simulink model "MX_Gateway"  (Simulink 9.0)
settings: Solver=FixedStepDiscrete  StartTime=0.0  StopTime=100  FixedStep=0.01  RelTol=1e-3
values from neighbouring files (symbols this model uses):
  ACTIVE = Bobcat.Parameter; CreateDefine( ACTIVE                         ,1                          ,'Define' ,'defines.h' )    [defines.m]
  ACTIVE_MODE_ONE = Bobcat.Parameter; CreateDefine( ACTIVE_MODE_ONE                ,19                         ,'Define' ,'defines.h' )    [defines.m]
  AUTHENTICATED = Bobcat.Parameter; CreateDefine( AUTHENTICATED                  ,1                          ,'Define' ,'defines.h' )    [defines.m]
  AUX_EMEA_CONTROLS = Bobcat.Parameter; CreateDefine( AUX_EMEA_CONTROLS  ,1   ,'Define' ,'defines.h' )    [enums.m]
  AUX_FLOW_HIGH = Bobcat.Parameter; CreateDefine( AUX_FLOW_HIGH           ,4  ,'Define' ,'defines.h' )    [enums.m]
  AUX_FLOW_INACTIVE = Bobcat.Parameter; CreateDefine( AUX_FLOW_INACTIVE       ,0  ,'Define' ,'defines.h' )    [enums.m]
  AUX_FLOW_LOW = Bobcat.Parameter; CreateDefine( AUX_FLOW_LOW            ,1  ,'Define' ,'defines.h' )    [enums.m]
  AUX_FLOW_MEDIUM = Bobcat.Parameter; CreateDefine( AUX_FLOW_MEDIUM         ,2  ,'Define' ,'defines.h' )    [enums.m]
  AUX_FLOW_STANDARD = Bobcat.Parameter; CreateDefine( AUX_FLOW_STANDARD       ,3  ,'Define' ,'defines.h' )    [enums.m]
  AUX_NON_FUNCTIONAL = Bobcat.Parameter; CreateDefine( AUX_NON_FUNCTIONAL ,255 ,'Define' ,'defines.h' )    [enums.m]
  BASE_ACTIVE = Bobcat.Parameter; CreateDefine( BASE_ACTIVE                    ,253                        ,'Define' ,'defines.h' )    [defines.m]
  BASE_DETENT = Bobcat.Parameter; CreateDefine( BASE_DETENT                    ,1                          ,'Define' ,'defines.h' )    [defines.m]
  BEEP_NONE = Bobcat.Parameter; CreateDefine( BEEP_NONE                      ,255                        ,'Define' ,'defines.h' )    [defines.m]
  CD_AIR_FILTER_DEBOUNCE_NORMAL = Bobcat.Parameter; CreateAliasCD( CD_AIR_FILTER_DEBOUNCE_NORMAL                        ,uint16(500)                ,'ConstantDataPointer' ,'CONSTANT_DATA' ,'AIR_FILTER_DEBOUNCE_NORMAL')    [cdata.m]    (also in hc12_cdata.m; m4_cdata.m)
  CD_AIR_FILTER_DEBOUNCE_NOT_CONNECTED = Bobcat.Parameter; CreateAliasCD( CD_AIR_FILTER_DEBOUNCE_NOT_CONNECTED                 ,uint16(3000)               ,'ConstantDataPointer' ,'CONSTANT_DATA' ,'AIR_FILTER_DEBOUNCE_NOT_CONNECTED')    [cdata.m]    (also in hc12_cdata.m; m4_cdata.m)
  CD_AIR_FILTER_DEBOUNCE_PLUGGED = Bobcat.Parameter; CreateAliasCD( CD_AIR_FILTER_DEBOUNCE_PLUGGED                       ,uint16(3000)               ,'ConstantDataPointer' ,'CONSTANT_DATA' ,'AIR_FILTER_DEBOUNCE_PLUGGED')    [cdata.m]    (also in hc12_cdata.m; m4_cdata.m)
  CD_AIR_FILTER_FUNCTION = Bobcat.Parameter; CreateAliasCD( CD_AIR_FILTER_FUNCTION                               ,uint8(255)                 ,'ConstantDataPointer' ,'CONSTANT_DATA' ,'AIR_FILTER_FUNCTION')    [cdata.m]    (also in hc12_cdata.m; m4_cdata.m)
  CD_AIR_FILTER_PLUGGED_WEIGHT = Bobcat.Parameter; CreateAliasCD( CD_AIR_FILTER_PLUGGED_WEIGHT                         ,uint8(2)                   ,'ConstantDataPointer' ,'CONSTANT_DATA' ,'AIR_FILTER_PLUGGED_WEIGHT')    [cdata.m]    (also in hc12_cdata.m; m4_cdata.m)
  CD_AIR_FILTER_UNPLUGGED_WEIGHT = Bobcat.Parameter; CreateAliasCD( CD_AIR_FILTER_UNPLUGGED_WEIGHT                       ,uint8(1)                   ,'ConstantDataPointer' ,'CONSTANT_DATA' ,'AIR_FILTER_UNPLUGGED_WEIGHT')    [cdata.m]    (also in hc12_cdata.m; m4_cdata.m)
  CD_AUTOIDLE_ARMBAR_DELAY = Bobcat.Parameter; CreateAliasCD( CD_AUTOIDLE_ARMBAR_DELAY                             ,uint8(2)                   ,'ConstantDataPointer' ,'CONSTANT_DATA' ,'AUTOIDLE_ARMBAR_DELAY')    [cdata.m]    (also in hc12_cdata.m; m4_cdata.m)
  CD_AUTOIDLE_FUNCTION = Bobcat.Parameter; CreateAliasCD( CD_AUTOIDLE_FUNCTION                                 ,uint8(255)                 ,'ConstantDataPointer' ,'CONSTANT_DATA' ,'AUTOIDLE_FUNCTION')    [cdata.m]    (also in hc12_cdata.m; m4_cdata.m)
  CD_AUTOIDLE_IDLE_DELAY = Bobcat.Parameter; CreateAliasCD( CD_AUTOIDLE_IDLE_DELAY                               ,uint8(40)                  ,'ConstantDataPointer' ,'CONSTANT_DATA' ,'AUTOIDLE_IDLE_DELAY')    [cdata.m]    (also in hc12_cdata.m; m4_cdata.m)
  CD_AUTOIDLE_LOW_IDLE_START = Bobcat.Parameter; CreateAliasCD( CD_AUTOIDLE_LOW_IDLE_START                           ,uint8(255)                 ,'ConstantDataPointer' ,'CONSTANT_DATA' ,'AUTOIDLE_LOW_IDLE_START')    [cdata.m]    (also in hc12_cdata.m; m4_cdata.m)
  CD_AUTOIDLE_PRESSURE_OFF = Bobcat.Parameter; CreateAliasCD( CD_AUTOIDLE_PRESSURE_OFF                             ,uint16(300)                ,'ConstantDataPointer' ,'CONSTANT_DATA' ,'AUTOIDLE_PRESSURE_OFF')    [cdata.m]    (also in hc12_cdata.m; m4_cdata.m)
  CD_AUTOIDLE_PRESSURE_ON = Bobcat.Parameter; CreateAliasCD( CD_AUTOIDLE_PRESSURE_ON                              ,uint16(270)                ,'ConstantDataPointer' ,'CONSTANT_DATA' ,'AUTOIDLE_PRESSURE_ON')    [cdata.m]    (also in hc12_cdata.m; m4_cdata.m)
  CD_AUTOSHIFT_FUNCTION = Bobcat.Parameter; CreateAliasCD( CD_AUTOSHIFT_FUNCTION                                ,uint8(255)                 ,'ConstantDataPointer' ,'CONSTANT_DATA' ,'AUTOSHIFT_FUNCTION')    [cdata.m]    (also in hc12_cdata.m; m4_cdata.m)
  CD_AUTOSHIFT_PHIGH1 = Bobcat.Parameter; CreateAliasCD( CD_AUTOSHIFT_PHIGH1                                  ,uint16(205)                ,'ConstantDataPointer' ,'CONSTANT_DATA' ,'AUTOSHIFT_PHIGH1')    [cdata.m]    (also in hc12_cdata.m; m4_cdata.m)
  CD_AUTOSHIFT_PHIGH2 = Bobcat.Parameter; CreateAliasCD( CD_AUTOSHIFT_PHIGH2                                  ,uint16(193)                ,'ConstantDataPointer' ,'CONSTANT_DATA' ,'AUTOSHIFT_PHIGH2')    [cdata.m]    (also in hc12_cdata.m; m4_cdata.m)
  CD_AUTOSHIFT_PHIGH_TEMP1 = Bobcat.Parameter; CreateAliasCD( CD_AUTOSHIFT_PHIGH_TEMP1                             ,int16(70)                  ,'ConstantDataPointer' ,'CONSTANT_DATA' ,'AUTOSHIFT_PHIGH_TEMP1')    [cdata.m]    (also in hc12_cdata.m; m4_cdata.m)
  CD_AUTOSHIFT_PHIGH_TEMP2 = Bobcat.Parameter; CreateAliasCD( CD_AUTOSHIFT_PHIGH_TEMP2                             ,int16(160)                 ,'ConstantDataPointer' ,'CONSTANT_DATA' ,'AUTOSHIFT_PHIGH_TEMP2')    [cdata.m]    (also in hc12_cdata.m; m4_cdata.m)
  CD_AUTOSHIFT_PLOW1 = Bobcat.Parameter; CreateAliasCD( CD_AUTOSHIFT_PLOW1                                   ,uint16(130)                ,'ConstantDataPointer' ,'CONSTANT_DATA' ,'AUTOSHIFT_PLOW1')    [cdata.m]    (also in hc12_cdata.m; m4_cdata.m)
  CD_AUTOSHIFT_PLOW2 = Bobcat.Parameter; CreateAliasCD( CD_AUTOSHIFT_PLOW2                                   ,uint16(100)                ,'ConstantDataPointer' ,'CONSTANT_DATA' ,'AUTOSHIFT_PLOW2')    [cdata.m]    (also in hc12_cdata.m; m4_cdata.m)
  CD_AUTOSHIFT_PLOW_TEMP1 = Bobcat.Parameter; CreateAliasCD( CD_AUTOSHIFT_PLOW_TEMP1                              ,int16(20)                  ,'ConstantDataPointer' ,'CONSTANT_DATA' ,'AUTOSHIFT_PLOW_TEMP1')    [cdata.m]    (also in hc12_cdata.m; m4_cdata.m)
  CD_AUTOSHIFT_PLOW_TEMP2 = Bobcat.Parameter; CreateAliasCD( CD_AUTOSHIFT_PLOW_TEMP2                              ,int16(80)                  ,'ConstantDataPointer' ,'CONSTANT_DATA' ,'AUTOSHIFT_PLOW_TEMP2')    [cdata.m]    (also in hc12_cdata.m; m4_cdata.m)
  CD_AUTOSHIFT_TRAVEL_DELAY = Bobcat.Parameter; CreateAliasCD( CD_AUTOSHIFT_TRAVEL_DELAY                            ,uint8(32)                  ,'ConstantDataPointer' ,'CONSTANT_DATA' ,'AUTOSHIFT_TRAVEL_DELAY')    [cdata.m]    (also in hc12_cdata.m; m4_cdata.m)
  CD_AUX2_CRACKPOINT_CURRENT = Bobcat.Parameter; CreateAliasCD( CD_AUX2_CRACKPOINT_CURRENT                           ,fi(0,0,16,12,'hex','0c28') ,'ConstantDataPointer' ,'CONSTANT_DATA' ,'AUX2_CRACKPOINT_CURRENT')    [cdata.m]    (also in hc12_cdata.m; m4_cdata.m)
  CD_AUX2_FLOW_LOW_FUNCTION = Bobcat.Parameter; CreateAliasCD( CD_AUX2_FLOW_LOW_FUNCTION                            ,uint8(255)                 ,'ConstantDataPointer' ,'CONSTANT_DATA' ,'AUX2_FLOW_LOW_FUNCTION')    [cdata.m]    (also in hc12_cdata.m; m4_cdata.m)
  CD_AUX2_FLOW_MED_FUNCTION = Bobcat.Parameter; CreateAliasCD( CD_AUX2_FLOW_MED_FUNCTION                            ,uint8(255)                 ,'ConstantDataPointer' ,'CONSTANT_DATA' ,'AUX2_FLOW_MED_FUNCTION')    [cdata.m]    (also in hc12_cdata.m; m4_cdata.m)
  CD_AUX2_HI_FLOW_PERCENT = Bobcat.Parameter; CreateAliasCD( CD_AUX2_HI_FLOW_PERCENT                              ,fi(0,0,16,16,'hex','ffff') ,'ConstantDataPointer' ,'CONSTANT_DATA' ,'AUX2_HI_FLOW_PERCENT')    [cdata.m]    (also in hc12_cdata.m; m4_cdata.m)
  CD_AUX2_LOW_FLOW_PERCENT = Bobcat.Parameter; CreateAliasCD( CD_AUX2_LOW_FLOW_PERCENT                             ,fi(0,0,16,16,'hex','7333') ,'ConstantDataPointer' ,'CONSTANT_DATA' ,'AUX2_LOW_FLOW_PERCENT')    [cdata.m]    (also in hc12_cdata.m; m4_cdata.m)
  CD_AUX2_MAX_FLOW_CURRENT = Bobcat.Parameter; CreateAliasCD( CD_AUX2_MAX_FLOW_CURRENT                             ,fi(0,0,16,12,'hex','14cc') ,'ConstantDataPointer' ,'CONSTANT_DATA' ,'AUX2_MAX_FLOW_CURRENT')    [cdata.m]    (also in hc12_cdata.m; m4_cdata.m)
  CD_AUX2_MED_FLOW_PERCENT = Bobcat.Parameter; CreateAliasCD( CD_AUX2_MED_FLOW_PERCENT                             ,fi(0,0,16,16,'hex','9999') ,'ConstantDataPointer' ,'CONSTANT_DATA' ,'AUX2_MED_FLOW_PERCENT')    [cdata.m]    (also in hc12_cdata.m; m4_cdata.m)
  CD_AUX_CRACKPOINT_CURRENT = Bobcat.Parameter; CreateAliasCD( CD_AUX_CRACKPOINT_CURRENT                            ,fi(0,0,16,12,'hex','0000') ,'ConstantDataPointer' ,'CONSTANT_DATA' ,'AUX_CRACKPOINT_CURRENT')    [cdata.m]    (also in hc12_cdata.m; m4_cdata.m)
  CD_AUX_DETENT_FLASH_TIME = Bobcat.Parameter; CreateAliasCD( CD_AUX_DETENT_FLASH_TIME                             ,uint16(72)                 ,'ConstantDataPointer' ,'CONSTANT_DATA' ,'AUX_DETENT_FLASH_TIME')    [cdata.m]    (also in hc12_cdata.m; m4_cdata.m)
  CD_AUX_DETENT_HOLD_TIME = Bobcat.Parameter; CreateAliasCD( CD_AUX_DETENT_HOLD_TIME                              ,uint16(100)                ,'ConstantDataPointer' ,'CONSTANT_DATA' ,'AUX_DETENT_HOLD_TIME')    [cdata.m]    (also in hc12_cdata.m; m4_cdata.m)
  CD_AUX_FULL_ON_AUX1 = Bobcat.Parameter; CreateAliasCD( CD_AUX_FULL_ON_AUX1                                  ,fi(0,0,16,12,'hex','10cd') ,'ConstantDataPointer' ,'CONSTANT_DATA' ,'AUX_FULL_ON_AUX1')    [cdata.m]    (also in hc12_cdata.m; m4_cdata.m)
  CD_AUX_FULL_ON_AUX2 = Bobcat.Parameter; CreateAliasCD( CD_AUX_FULL_ON_AUX2                                  ,fi(0,0,16,12,'hex','139d') ,'ConstantDataPointer' ,'CONSTANT_DATA' ,'AUX_FULL_ON_AUX2')    [cdata.m]    (also in hc12_cdata.m; m4_cdata.m)
  CD_AUX_FULL_ON_AUX3 = Bobcat.Parameter; CreateAliasCD( CD_AUX_FULL_ON_AUX3                                  ,fi(0,0,16,12,'hex','17d7') ,'ConstantDataPointer' ,'CONSTANT_DATA' ,'AUX_FULL_ON_AUX3')    [cdata.m]    (also in hc12_cdata.m; m4_cdata.m)
  CD_AUX_FUNCTION = Bobcat.Parameter; CreateAliasCD( CD_AUX_FUNCTION                                      ,uint8(0)                   ,'ConstantDataPointer' ,'CONSTANT_DATA' ,'AUX_FUNCTION')    [cdata.m]    (also in hc12_cdata.m; m4_cdata.m)
  CD_AUX_RATE_LIMIT_CRACKPOINT = Bobcat.Parameter; CreateAliasCD( CD_AUX_RATE_LIMIT_CRACKPOINT                         ,fi(0,0,16,12,'hex','0800') ,'ConstantDataPointer' ,'CONSTANT_DATA' ,'AUX_RATE_LIMIT_CRACKPOINT')    [cdata.m]    (also in hc12_cdata.m; m4_cdata.m)
  CD_AUX_RATE_LIMIT_CRACKPOINT_RATE = Bobcat.Parameter; CreateAliasCD( CD_AUX_RATE_LIMIT_CRACKPOINT_RATE                    ,fi(0,1,32,12,'int',102400) ,'ConstantDataPointer' ,'CONSTANT_DATA' ,'AUX_RATE_LIMIT_CRACKPOINT_RATE')    [cdata.m]    (also in hc12_cdata.m; m4_cdata.m)
  CD_AUX_RATE_LIMIT_FUNCTION = Bobcat.Parameter; CreateAliasCD( CD_AUX_RATE_LIMIT_FUNCTION                           ,uint8(255)                 ,'ConstantDataPointer' ,'CONSTANT_DATA' ,'AUX_RATE_LIMIT_FUNCTION')    [cdata.m]    (also in hc12_cdata.m; m4_cdata.m)
  CD_AUX_RATE_LIMIT_MAX_ENG_SPD = Bobcat.Parameter; CreateAliasCD( CD_AUX_RATE_LIMIT_MAX_ENG_SPD                        ,uint16(1600)               ,'ConstantDataPointer' ,'CONSTANT_DATA' ,'AUX_RATE_LIMIT_MAX_ENG_SPD')    [cdata.m]    (also in hc12_cdata.m; m4_cdata.m)
  CD_AUX_RATE_LIMIT_MIN_ENG_SPD = Bobcat.Parameter; CreateAliasCD( CD_AUX_RATE_LIMIT_MIN_ENG_SPD                        ,uint16(100)                ,'ConstantDataPointer' ,'CONSTANT_DATA' ,'AUX_RATE_LIMIT_MIN_ENG_SPD')    [cdata.m]    (also in hc12_cdata.m; m4_cdata.m)
  CD_AUX_RATE_LIMIT_NORMAL_RATE = Bobcat.Parameter; CreateAliasCD( CD_AUX_RATE_LIMIT_NORMAL_RATE                        ,fi(0,1,32,12,'int',2048)   ,'ConstantDataPointer' ,'CONSTANT_DATA' ,'AUX_RATE_LIMIT_NORMAL_RATE')    [cdata.m]    (also in hc12_cdata.m; m4_cdata.m)
  CD_AUXPOT_CAL_DEADBAND_HIGH = Bobcat.Parameter; CreateAliasCD( CD_AUXPOT_CAL_DEADBAND_HIGH                          ,uint16(574)                ,'ConstantDataPointer' ,'CONSTANT_DATA' ,'AUXPOT_CAL_DEADBAND_HIGH')    [cdata.m]    (also in hc12_cdata.m; m4_cdata.m)
  CD_AUXPOT_CAL_DEADBAND_LOW = Bobcat.Parameter; CreateAliasCD( CD_AUXPOT_CAL_DEADBAND_LOW                           ,uint16(424)                ,'ConstantDataPointer' ,'CONSTANT_DATA' ,'AUXPOT_CAL_DEADBAND_LOW')    [cdata.m]    (also in hc12_cdata.m; m4_cdata.m)
  CD_AUXPOT_CENTER_RATIO = Bobcat.Parameter; CreateAliasCD( CD_AUXPOT_CENTER_RATIO                               ,uint16(500)                ,'ConstantDataPointer' ,'CONSTANT_DATA' ,'AUXPOT_CENTER_RATIO')    [cdata.m]    (also in hc12_cdata.m; m4_cdata.m)
  CD_AUXPOT_DEADBAND = Bobcat.Parameter; CreateAliasCD( CD_AUXPOT_DEADBAND                                   ,uint8(38)                  ,'ConstantDataPointer' ,'CONSTANT_DATA' ,'AUXPOT_DEADBAND')    [cdata.m]    (also in hc12_cdata.m; m4_cdata.m)
  CD_AUXPOT_FUNCTION = Bobcat.Parameter; CreateAliasCD( CD_AUXPOT_FUNCTION                                   ,uint8(0)                   ,'ConstantDataPointer' ,'CONSTANT_DATA' ,'AUXPOT_FUNCTION')    [cdata.m]    (also in hc12_cdata.m; m4_cdata.m)
  CD_AUXPOT_RATIO_GAIN = Bobcat.Parameter; CreateAliasCD( CD_AUXPOT_RATIO_GAIN                                 ,uint16(1000)               ,'ConstantDataPointer' ,'CONSTANT_DATA' ,'AUXPOT_RATIO_GAIN')    [cdata.m]    (also in hc12_cdata.m; m4_cdata.m)
  CD_CAN_THROTTLE_FUNCTION = Bobcat.Parameter; CreateAliasCD( CD_CAN_THROTTLE_FUNCTION                             ,uint8(255)                 ,'ConstantDataPointer' ,'CONSTANT_DATA' ,'CAN_THROTTLE_FUNCTION')    [cdata.m]    (also in hc12_cdata.m; m4_cdata.m)
  CD_CAN_THROTTLE_REVERSE = Bobcat.Parameter; CreateAliasCD( CD_CAN_THROTTLE_REVERSE                              ,uint8(255)                 ,'ConstantDataPointer' ,'CONSTANT_DATA' ,'CAN_THROTTLE_REVERSE')    [cdata.m]    (also in hc12_cdata.m; m4_cdata.m)
  CD_CONSOLE_DEBOUNCE_ABSENT = Bobcat.Parameter; CreateAliasCD( CD_CONSOLE_DEBOUNCE_ABSENT                           ,uint16(12)                 ,'ConstantDataPointer' ,'CONSTANT_DATA' ,'CONSOLE_DEBOUNCE_ABSENT')    [cdata.m]    (also in hc12_cdata.m; m4_cdata.m)
  CD_CONSOLE_DEBOUNCE_OORH = Bobcat.Parameter; CreateAliasCD( CD_CONSOLE_DEBOUNCE_OORH                             ,uint16(12)                 ,'ConstantDataPointer' ,'CONSTANT_DATA' ,'CONSOLE_DEBOUNCE_OORH')    [cdata.m]    (also in hc12_cdata.m; m4_cdata.m)
  CD_CONSOLE_DEBOUNCE_OORL = Bobcat.Parameter; CreateAliasCD( CD_CONSOLE_DEBOUNCE_OORL                             ,uint16(12)                 ,'ConstantDataPointer' ,'CONSTANT_DATA' ,'CONSOLE_DEBOUNCE_OORL')    [cdata.m]    (also in hc12_cdata.m; m4_cdata.m)
  CD_CONSOLE_DEBOUNCE_PRESENT = Bobcat.Parameter; CreateAliasCD( CD_CONSOLE_DEBOUNCE_PRESENT                          ,uint16(12)                 ,'ConstantDataPointer' ,'CONSTANT_DATA' ,'CONSOLE_DEBOUNCE_PRESENT')    [cdata.m]    (also in hc12_cdata.m; m4_cdata.m)
  CD_CONSOLE_FUNCTION = Bobcat.Parameter; CreateAliasCD( CD_CONSOLE_FUNCTION                                  ,uint8(0)                   ,'ConstantDataPointer' ,'CONSTANT_DATA' ,'CONSOLE_FUNCTION')    [cdata.m]    (also in hc12_cdata.m; m4_cdata.m)
  CD_CONSOLE_OORH = Bobcat.Parameter; CreateAliasCD( CD_CONSOLE_OORH                                      ,uint16(740)                ,'ConstantDataPointer' ,'CONSTANT_DATA' ,'CONSOLE_OORH')    [cdata.m]    (also in hc12_cdata.m; m4_cdata.m)
  CD_CONSOLE_OORL = Bobcat.Parameter; CreateAliasCD( CD_CONSOLE_OORL                                      ,uint16(10)                 ,'ConstantDataPointer' ,'CONSTANT_DATA' ,'CONSOLE_OORL')    [cdata.m]    (also in hc12_cdata.m; m4_cdata.m)
  CD_CONSOLE_PRESENT_HIGH = Bobcat.Parameter; CreateAliasCD( CD_CONSOLE_PRESENT_HIGH                              ,uint16(515)                ,'ConstantDataPointer' ,'CONSTANT_DATA' ,'CONSOLE_PRESENT_HIGH')    [cdata.m]    (also in hc12_cdata.m; m4_cdata.m)
  CD_CONSOLE_PRESENT_LOW = Bobcat.Parameter; CreateAliasCD( CD_CONSOLE_PRESENT_LOW                               ,uint16(375)                ,'ConstantDataPointer' ,'CONSTANT_DATA' ,'CONSOLE_PRESENT_LOW')    [cdata.m]    (also in hc12_cdata.m; m4_cdata.m)
  CD_DELUXE_GEN5_5_FUNCTION = Bobcat.Parameter; CreateAliasCD( CD_DELUXE_GEN5_5_FUNCTION                            ,uint8(255)                 ,'ConstantDataPointer' ,'CONSTANT_DATA' ,'DELUXE_GEN5_5_FUNCTION')    [cdata.m]    (also in hc12_cdata.m; m4_cdata.m)
  CD_DELUXE_GEN5_FUNCTION = Bobcat.Parameter; CreateAliasCD( CD_DELUXE_GEN5_FUNCTION                              ,uint8(255)                 ,'ConstantDataPointer' ,'CONSTANT_DATA' ,'DELUXE_GEN5_FUNCTION')    [cdata.m]    (also in hc12_cdata.m; m4_cdata.m)
  CD_DERATE_ENGINE_SPEED_DEBOUNCE_HIGH = Bobcat.Parameter; CreateAliasCD( CD_DERATE_ENGINE_SPEED_DEBOUNCE_HIGH                 ,uint16(200)                ,'ConstantDataPointer' ,'CONSTANT_DATA' ,'DERATE_ENGINE_SPEED_DEBOUNCE_HIGH')    [cdata.m]    (also in hc12_cdata.m; m4_cdata.m)
  CD_DERATE_ENGINE_SPEED_DEBOUNCE_SHUTDOWN = Bobcat.Parameter; CreateAliasCD( CD_DERATE_ENGINE_SPEED_DEBOUNCE_SHUTDOWN             ,uint16(1000)               ,'ConstantDataPointer' ,'CONSTANT_DATA' ,'DERATE_ENGINE_SPEED_DEBOUNCE_SHUTDOWN')    [cdata.m]    (also in hc12_cdata.m; m4_cdata.m)
  CD_DERATE_ENGINE_SPEED_HIGH_OFFSET = Bobcat.Parameter; CreateAliasCD( CD_DERATE_ENGINE_SPEED_HIGH_OFFSET                   ,uint16(200)                ,'ConstantDataPointer' ,'CONSTANT_DATA' ,'DERATE_ENGINE_SPEED_HIGH_OFFSET')    [cdata.m]    (also in hc12_cdata.m; m4_cdata.m)
  CD_DERATE_METHOD = Bobcat.Parameter; CreateAliasCD( CD_DERATE_METHOD                                     ,uint8(0)                   ,'ConstantDataPointer' ,'CONSTANT_DATA' ,'DERATE_METHOD')    [cdata.m]    (also in hc12_cdata.m; m4_cdata.m)
  CD_DUMP_VALVE_FUNCTION = Bobcat.Parameter; CreateAliasCD( CD_DUMP_VALVE_FUNCTION                               ,uint8(255)                 ,'ConstantDataPointer' ,'CONSTANT_DATA' ,'DUMP_VALVE_FUNCTION')    [cdata.m]    (also in hc12_cdata.m; m4_cdata.m)
  CD_DUMP_VALVE_ON_CURRENT = Bobcat.Parameter; CreateAliasCD( CD_DUMP_VALVE_ON_CURRENT                             ,fi(0,0,16,12,'hex','ffff') ,'ConstantDataPointer' ,'CONSTANT_DATA' ,'DUMP_VALVE_ON_CURRENT')    [cdata.m]    (also in hc12_cdata.m; m4_cdata.m)
  CD_ECO_MODE_THROTTLE_REDUCTION = Bobcat.Parameter; CreateAliasCD( CD_ECO_MODE_THROTTLE_REDUCTION                       ,uint16(850)                ,'ConstantDataPointer' ,'CONSTANT_DATA' ,'ECO_MODE_THROTTLE_REDUCTION')    [cdata.m]    (also in hc12_cdata.m; m4_cdata.m)
  CD_ECT_FILTER_GAIN = Bobcat.Parameter; CreateAliasCD( CD_ECT_FILTER_GAIN                                   ,uint8(6)                   ,'ConstantDataPointer' ,'CONSTANT_DATA' ,'ECT_FILTER_GAIN')    [cdata.m]    (also in hc12_cdata.m; m4_cdata.m)
  CD_ECU_FUNCTION = Bobcat.Parameter; CreateAliasCD( CD_ECU_FUNCTION                                      ,uint8(255)                 ,'ConstantDataPointer' ,'CONSTANT_DATA' ,'ECU_FUNCTION')    [cdata.m]    (also in hc12_cdata.m; m4_cdata.m)
  CD_ECU_HOLD_DEBOUNCE_ERROR_ON = Bobcat.Parameter; CreateAliasCD( CD_ECU_HOLD_DEBOUNCE_ERROR_ON                        ,uint16(20)                 ,'ConstantDataPointer' ,'CONSTANT_DATA' ,'ECU_HOLD_DEBOUNCE_ERROR_ON')    [cdata.m]    (also in hc12_cdata.m; m4_cdata.m)
  CD_EMEA_CONTROLS_HOLD_TIME = Bobcat.Parameter; CreateAliasCD( CD_EMEA_CONTROLS_HOLD_TIME                           ,uint16(30)                 ,'ConstantDataPointer' ,'CONSTANT_DATA' ,'EMEA_CONTROLS_HOLD_TIME')    [cdata.m]    (also in hc12_cdata.m; m4_cdata.m)
  CD_EMEA_CONTROLS_OPTION_A = Bobcat.Parameter; CreateAliasCD( CD_EMEA_CONTROLS_OPTION_A                            ,uint8(255)                 ,'ConstantDataPointer' ,'CONSTANT_DATA' ,'EMEA_CONTROLS_OPTION_A')    [cdata.m]    (also in hc12_cdata.m; m4_cdata.m)
  CD_EMEA_CONTROLS_OPTION_C = Bobcat.Parameter; CreateAliasCD( CD_EMEA_CONTROLS_OPTION_C                            ,uint8(255)                 ,'ConstantDataPointer' ,'CONSTANT_DATA' ,'EMEA_CONTROLS_OPTION_C')    [cdata.m]    (also in hc12_cdata.m; m4_cdata.m)
  CD_EMEA_CONTROLS_REVERSE = Bobcat.Parameter; CreateAliasCD( CD_EMEA_CONTROLS_REVERSE                             ,uint8(255)                 ,'ConstantDataPointer' ,'CONSTANT_DATA' ,'EMEA_CONTROLS_REVERSE')    [cdata.m]    (also in hc12_cdata.m; m4_cdata.m)
  CD_EMEA_ELECTRIC_VERSION = Bobcat.Parameter; CreateAliasCD( CD_EMEA_ELECTRIC_VERSION                             ,uint8(0)                   ,'ConstantDataPointer' ,'CONSTANT_DATA' ,'EMEA_ELECTRIC_VERSION')    [cdata.m]    (also in hc12_cdata.m; m4_cdata.m)
  CD_EMEA_POT_CAL_DEADBAND_HIGH = Bobcat.Parameter; CreateAliasCD( CD_EMEA_POT_CAL_DEADBAND_HIGH                        ,uint16(574)                ,'ConstantDataPointer' ,'CONSTANT_DATA' ,'EMEA_POT_CAL_DEADBAND_HIGH')    [cdata.m]    (also in hc12_cdata.m; m4_cdata.m)
  CD_EMEA_POT_CAL_DEADBAND_LOW = Bobcat.Parameter; CreateAliasCD( CD_EMEA_POT_CAL_DEADBAND_LOW                         ,uint16(424)                ,'ConstantDataPointer' ,'CONSTANT_DATA' ,'EMEA_POT_CAL_DEADBAND_LOW')    [cdata.m]    (also in hc12_cdata.m; m4_cdata.m)
  CD_ENGINE_RPM_FACTOR = Bobcat.Parameter; CreateAliasCD( CD_ENGINE_RPM_FACTOR                                 ,uint8(35)                  ,'ConstantDataPointer' ,'CONSTANT_DATA' ,'ENGINE_RPM_FACTOR')    [cdata.m]    (also in hc12_cdata.m; m4_cdata.m)
  CD_ENGINE_SPEED_FILTER_FAST_VALUE = Bobcat.Parameter; CreateAliasCD( CD_ENGINE_SPEED_FILTER_FAST_VALUE                    ,uint8(1)                   ,'ConstantDataPointer' ,'CONSTANT_DATA' ,'ENGINE_SPEED_FILTER_FAST_VALUE')    [cdata.m]    (also in hc12_cdata.m; m4_cdata.m)
  CD_ENGINE_SPEED_FILTER_SLOW_VALUE = Bobcat.Parameter; CreateAliasCD( CD_ENGINE_SPEED_FILTER_SLOW_VALUE                    ,uint8(4)                   ,'ConstantDataPointer' ,'CONSTANT_DATA' ,'ENGINE_SPEED_FILTER_SLOW_VALUE')    [cdata.m]    (also in hc12_cdata.m; m4_cdata.m)
  CD_ENGINE_SPEED_RUNNING = Bobcat.Parameter; CreateAliasCD( CD_ENGINE_SPEED_RUNNING                              ,uint16(800)                ,'ConstantDataPointer' ,'CONSTANT_DATA' ,'ENGINE_SPEED_RUNNING')    [cdata.m]    (also in hc12_cdata.m; m4_cdata.m)
  CD_ENGINE_START_DELAY_DEBOUNCE_RPM_NO_SIGNAL = Bobcat.Parameter; CreateAliasCD( CD_ENGINE_START_DELAY_DEBOUNCE_RPM_NO_SIGNAL         ,uint16(0)                  ,'ConstantDataPointer' ,'CONSTANT_DATA' ,'ENGINE_START_DELAY_DEBOUNCE_RPM_NO_SIGNAL')    [cdata.m]    (also in hc12_cdata.m; m4_cdata.m)
  CD_ENGINE_START_DELAY_OFF_TIME = Bobcat.Parameter; CreateAliasCD( CD_ENGINE_START_DELAY_OFF_TIME                       ,uint16(0)                  ,'ConstantDataPointer' ,'CONSTANT_DATA' ,'ENGINE_START_DELAY_OFF_TIME')    [cdata.m]    (also in hc12_cdata.m; m4_cdata.m)
  CD_ENGINE_START_DELAY_RPM_NO_SIGNAL = Bobcat.Parameter; CreateAliasCD( CD_ENGINE_START_DELAY_RPM_NO_SIGNAL                  ,uint16(0)                  ,'ConstantDataPointer' ,'CONSTANT_DATA' ,'ENGINE_START_DELAY_RPM_NO_SIGNAL')    [cdata.m]    (also in hc12_cdata.m; m4_cdata.m)
  CD_EOP_DEBOUNCE_EXTREMELY_LOW = Bobcat.Parameter; CreateAliasCD( CD_EOP_DEBOUNCE_EXTREMELY_LOW                        ,uint16(50)                 ,'ConstantDataPointer' ,'CONSTANT_DATA' ,'EOP_DEBOUNCE_EXTREMELY_LOW')    [cdata.m]    (also in hc12_cdata.m; m4_cdata.m)
  CD_EOP_DEBOUNCE_NORMAL = Bobcat.Parameter; CreateAliasCD( CD_EOP_DEBOUNCE_NORMAL                               ,uint16(50)                 ,'ConstantDataPointer' ,'CONSTANT_DATA' ,'EOP_DEBOUNCE_NORMAL')    [cdata.m]    (also in hc12_cdata.m; m4_cdata.m)
  CD_EOP_DEBOUNCE_SHUTDOWN = Bobcat.Parameter; CreateAliasCD( CD_EOP_DEBOUNCE_SHUTDOWN                             ,uint16(100)                ,'ConstantDataPointer' ,'CONSTANT_DATA' ,'EOP_DEBOUNCE_SHUTDOWN')    [cdata.m]    (also in hc12_cdata.m; m4_cdata.m)
  CD_EOP_FILTER_VALUE = Bobcat.Parameter; CreateAliasCD( CD_EOP_FILTER_VALUE                                  ,uint8(3)                   ,'ConstantDataPointer' ,'CONSTANT_DATA' ,'EOP_FILTER_VALUE')    [cdata.m]    (also in hc12_cdata.m; m4_cdata.m)
  CD_EOP_FUNCTION = Bobcat.Parameter; CreateAliasCD( CD_EOP_FUNCTION                                      ,uint8(1)                   ,'ConstantDataPointer' ,'CONSTANT_DATA' ,'EOP_FUNCTION')    [cdata.m]    (also in hc12_cdata.m; m4_cdata.m)
  CD_EOP_SWITCH_GAUGE_VALUE = Bobcat.Parameter; CreateAliasCD( CD_EOP_SWITCH_GAUGE_VALUE                            ,uint16(125)                ,'ConstantDataPointer' ,'CONSTANT_DATA' ,'EOP_SWITCH_GAUGE_VALUE')    [cdata.m]    (also in hc12_cdata.m; m4_cdata.m)
  CD_EOP_SWITCH_LOW = Bobcat.Parameter; CreateAliasCD( CD_EOP_SWITCH_LOW                                    ,uint16(50)                 ,'ConstantDataPointer' ,'CONSTANT_DATA' ,'EOP_SWITCH_LOW')    [cdata.m]    (also in hc12_cdata.m; m4_cdata.m)
  CD_EOP_SWITCH_PRESSURE_VALUE = Bobcat.Parameter; CreateAliasCD( CD_EOP_SWITCH_PRESSURE_VALUE                         ,uint16(40)                 ,'ConstantDataPointer' ,'CONSTANT_DATA' ,'EOP_SWITCH_PRESSURE_VALUE')    [cdata.m]    (also in hc12_cdata.m; m4_cdata.m)
  CD_EOT_DEBOUNCE_EXTREMELY_LOW = Bobcat.Parameter; CreateAliasCD( CD_EOT_DEBOUNCE_EXTREMELY_LOW                        ,uint16(2)                  ,'ConstantDataPointer' ,'CONSTANT_DATA' ,'EOT_DEBOUNCE_EXTREMELY_LOW')    [cdata.m]    (also in hc12_cdata.m; m4_cdata.m)
  CD_EOT_DEBOUNCE_LOW = Bobcat.Parameter; CreateAliasCD( CD_EOT_DEBOUNCE_LOW                                  ,uint16(2)                  ,'ConstantDataPointer' ,'CONSTANT_DATA' ,'EOT_DEBOUNCE_LOW')    [cdata.m]    (also in hc12_cdata.m; m4_cdata.m)
  CD_EOT_DEBOUNCE_NORMAL = Bobcat.Parameter; CreateAliasCD( CD_EOT_DEBOUNCE_NORMAL                               ,uint16(2)                  ,'ConstantDataPointer' ,'CONSTANT_DATA' ,'EOT_DEBOUNCE_NORMAL')    [cdata.m]    (also in hc12_cdata.m; m4_cdata.m)
  CD_EOT_EXTREMELY_LOW = Bobcat.Parameter; CreateAliasCD( CD_EOT_EXTREMELY_LOW                                 ,uint16(81)                 ,'ConstantDataPointer' ,'CONSTANT_DATA' ,'EOT_EXTREMELY_LOW')    [cdata.m]    (also in hc12_cdata.m; m4_cdata.m)
  CD_EOT_FUNCTION = Bobcat.Parameter; CreateAliasCD( CD_EOT_FUNCTION                                      ,uint8(255)                 ,'ConstantDataPointer' ,'CONSTANT_DATA' ,'EOT_FUNCTION')    [cdata.m]    (also in hc12_cdata.m; m4_cdata.m)
  CD_EOT_LOW = Bobcat.Parameter; CreateAliasCD( CD_EOT_LOW                                           ,uint16(90)                 ,'ConstantDataPointer' ,'CONSTANT_DATA' ,'EOT_LOW')    [cdata.m]    (also in hc12_cdata.m; m4_cdata.m)
  CD_EOT_TEMPERATURE_HYSTERESIS = Bobcat.Parameter; CreateAliasCD( CD_EOT_TEMPERATURE_HYSTERESIS                        ,uint16(2)                  ,'ConstantDataPointer' ,'CONSTANT_DATA' ,'EOT_TEMPERATURE_HYSTERESIS')    [cdata.m]    (also in hc12_cdata.m; m4_cdata.m)
  CD_FAST_TRAVEL_FUNCTION = Bobcat.Parameter; CreateAliasCD( CD_FAST_TRAVEL_FUNCTION                              ,uint8(255)                 ,'ConstantDataPointer' ,'CONSTANT_DATA' ,'FAST_TRAVEL_FUNCTION')    [cdata.m]    (also in hc12_cdata.m; m4_cdata.m)
  CD_FAST_TRAVEL_THROTTLE_DELAY = Bobcat.Parameter; CreateAliasCD( CD_FAST_TRAVEL_THROTTLE_DELAY                        ,uint16(10)                 ,'ConstantDataPointer' ,'CONSTANT_DATA' ,'FAST_TRAVEL_THROTTLE_DELAY')    [cdata.m]    (also in hc12_cdata.m; m4_cdata.m)
  CD_FAST_TRAVEL_THROTTLE_LIMIT = Bobcat.Parameter; CreateAliasCD( CD_FAST_TRAVEL_THROTTLE_LIMIT                        ,uint16(1000)               ,'ConstantDataPointer' ,'CONSTANT_DATA' ,'FAST_TRAVEL_THROTTLE_LIMIT')    [cdata.m]    (also in hc12_cdata.m; m4_cdata.m)
  CD_FLYWHEEL_TEETH = Bobcat.Parameter; CreateAliasCD( CD_FLYWHEEL_TEETH                                    ,uint8(83)                  ,'ConstantDataPointer' ,'CONSTANT_DATA' ,'FLYWHEEL_TEETH')    [cdata.m]    (also in hc12_cdata.m; m4_cdata.m)
  CD_FUEL_LEVEL_FILTER_FAST_VALUE = Bobcat.Parameter; CreateAliasCD( CD_FUEL_LEVEL_FILTER_FAST_VALUE                      ,uint8(5)                   ,'ConstantDataPointer' ,'CONSTANT_DATA' ,'FUEL_LEVEL_FILTER_FAST_VALUE')    [cdata.m]    (also in hc12_cdata.m; m4_cdata.m)
  CD_FUEL_LEVEL_FILTER_SLOW_VALUE = Bobcat.Parameter; CreateAliasCD( CD_FUEL_LEVEL_FILTER_SLOW_VALUE                      ,uint8(10)                  ,'ConstantDataPointer' ,'CONSTANT_DATA' ,'FUEL_LEVEL_FILTER_SLOW_VALUE')    [cdata.m]    (also in hc12_cdata.m; m4_cdata.m)
  CD_FUEL_PRIMARY_FEEDBACK_FUNCTION = Bobcat.Parameter; CreateAliasCD( CD_FUEL_PRIMARY_FEEDBACK_FUNCTION                    ,uint8(0)                   ,'ConstantDataPointer' ,'CONSTANT_DATA' ,'FUEL_PRIMARY_FEEDBACK_FUNCTION')    [cdata.m]    (also in hc12_cdata.m; m4_cdata.m)
  ... (+246 more)
undefined symbols (not defined in the file or in its folder): sigbuilder_block
undefined symbols (not defined in the file): CD_FUEL_RESOLUTION_TIMER
libraries: simulink x186, MX_Library x107
(part 6 of 48)

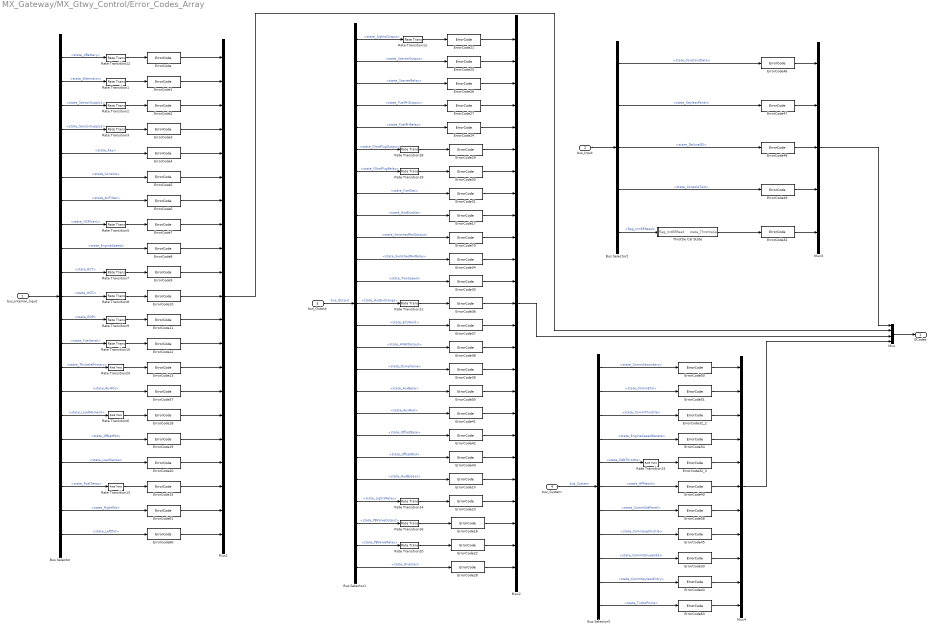
== system MX_Gateway/MX_Gtwy_Control/Error_Codes_Array ==
  bus_Internal_Input : Inport
  bus_Input : Inport  Port=2
  bus_Output : Inport  Port=3
  bus_System : Inport  Port=4
  Bus Selector : BusSelector  OutputSignals=state_VBattery,state_Alternator,state_SensorSupply1,state_SensorSupply2,state_Key,state_Console,state_AirFilter,state_HCFilter1,state_EngineSpeed,state_ECT,state_HOT,state_EOP,state_FuelLevel,state_ThrottlePrimary,state_AuxPot,state_LoadMoment,state_OffsetPot,state_LoadSense,state_FuelTemp,state_RightPot,state_LeftPot
  Bus Selector1 : BusSelector  OutputSignals=state_LightsOutput,state_StarterOutput,state_StarterRelay,state_FuelPriOutput,state_FuelPriRelay,state_GlowPlugOutput,state_GlowPlugRelay,state_FuelSec,state_HydEnable,state_SwitchedPwrOutput,state_SwitchedPwrRelay,state_TwoSpeed,state_HydExchange,state_ECUHold,state_HVACOutput,state_DumpValve,state_AuxBase,state_AuxRod,state_OffsetBase,state_OffsetRod,state_HydBypass,state_LightsRelay,state_PBValveOutput,state_PBValveRelay,state_Diverter
  Bus Selector2 : BusSelector  OutputSignals=bus_Controller.state_ConstantData,bus_CAN.bus_Keypad.state_KeylessPanel,bus_CAN.bus_DeluxeG5.state_DeluxeG5,bus_Controller.state_ConsoleTest,bus_Controller.flag_InitEERead
  Bus Selector3 : BusSelector  OutputSignals=state_CommSecondary,state_CommECU,state_CommThrottle,state_EngineSpeedDerate,state_CANThrottle,state_HPMatch,state_CommStdPanel,state_CommJogShuttle,state_CommDeluxeG55,state_CommKeylessEntry,state_TurboPrime
  ErrorCode : MX_Library/ErrorCode  FUNCTION=FN_BATTERY_VOLTAGE
      settings: AttributesFormatString=FUNCTION=%<FUNCTION>
  ErrorCode1 : MX_Library/ErrorCode  FUNCTION=FN_ALTERNATOR_VOLTAGE
      settings: AttributesFormatString=FUNCTION=%<FUNCTION>
  ErrorCode10 : MX_Library/ErrorCode  FUNCTION=FN_HYDRAULIC_OIL_TEMPERATURE
      settings: AttributesFormatString=FUNCTION=%<FUNCTION>
  ErrorCode11 : MX_Library/ErrorCode  FUNCTION=FN_ENGINE_OIL_PRESSURE
      settings: AttributesFormatString=FUNCTION=%<FUNCTION>
  ErrorCode12 : MX_Library/ErrorCode  FUNCTION=FN_FUEL_LEVEL
      settings: AttributesFormatString=FUNCTION=%<FUNCTION>
  ErrorCode13 : MX_Library/ErrorCode  FUNCTION=FN_THROTTLE_PRIMARY
      settings: AttributesFormatString=FUNCTION=%<FUNCTION>
  ErrorCode14 : MX_Library/ErrorCode  FUNCTION=FN_HYD_BYPASS
      settings: AttributesFormatString=FUNCTION=%<FUNCTION>
  ErrorCode15 : MX_Library/ErrorCode  FUNCTION=FN_FUEL_TEMP
      settings: AttributesFormatString=FUNCTION=%<FUNCTION>
  ErrorCode16 : MX_Library/ErrorCode  FUNCTION=FN_PBVALVE_OUTPUT
      settings: AttributesFormatString=FUNCTION=%<FUNCTION>
  ErrorCode17 : MX_Library/ErrorCode  FUNCTION=FN_HYD_ENABLE
      settings: AttributesFormatString=FUNCTION=%<FUNCTION>
  ErrorCode18 : MX_Library/ErrorCode  FUNCTION=FN_LOAD_MOMENT
      settings: AttributesFormatString=FUNCTION=%<FUNCTION>
  ErrorCode19 : MX_Library/ErrorCode  FUNCTION=FN_OFFSET_CONTROL
      settings: AttributesFormatString=FUNCTION=%<FUNCTION>
  ErrorCode2 : MX_Library/ErrorCode  FUNCTION=FN_SENSOR_SUPPLY_1
      settings: AttributesFormatString=FUNCTION=%<FUNCTION>
  ErrorCode20 : MX_Library/ErrorCode  FUNCTION=FN_LOAD_SENSE
      settings: AttributesFormatString=FUNCTION=%<FUNCTION>
  ErrorCode21 : MX_Library/ErrorCode  FUNCTION=FN_FRONT_LIGHT_OUTPUT
      settings: AttributesFormatString=FUNCTION=%<FUNCTION>
  ErrorCode22 : MX_Library/ErrorCode  FUNCTION=FN_PBVALVE_RELAY
      settings: AttributesFormatString=FUNCTION=%<FUNCTION>
  ErrorCode23 : MX_Library/ErrorCode  FUNCTION=FN_FRONT_LIGHT_RELAY
      settings: AttributesFormatString=FUNCTION=%<FUNCTION>
  ErrorCode24 : MX_Library/ErrorCode  FUNCTION=FN_FUEL_PRIMARY_RELAY
      settings: AttributesFormatString=FUNCTION=%<FUNCTION>
  ErrorCode25 : MX_Library/ErrorCode  FUNCTION=FN_STARTER_OUTPUT
      settings: AttributesFormatString=FUNCTION=%<FUNCTION>
  ErrorCode26 : MX_Library/ErrorCode  FUNCTION=FN_STARTER_RELAY
      settings: AttributesFormatString=FUNCTION=%<FUNCTION>
  ErrorCode27 : MX_Library/ErrorCode  FUNCTION=FN_FUEL_PRIMARY_OUTPUT
      settings: AttributesFormatString=FUNCTION=%<FUNCTION>
  ErrorCode28 : MX_Library/ErrorCode  FUNCTION=FN_DIVERTER
      settings: AttributesFormatString=FUNCTION=%<FUNCTION>
  ErrorCode29 : MX_Library/ErrorCode  FUNCTION=FN_GLOW_PLUG_OUTPUT
      settings: AttributesFormatString=FUNCTION=%<FUNCTION>
  ErrorCode3 : MX_Library/ErrorCode  FUNCTION=FN_SENSOR_SUPPLY_2
      settings: AttributesFormatString=FUNCTION=%<FUNCTION>
  ErrorCode30 : MX_Library/ErrorCode  FUNCTION=FN_GLOW_PLUG_RELAY
      settings: AttributesFormatString=FUNCTION=%<FUNCTION>
  ErrorCode31 : MX_Library/ErrorCode  FUNCTION=FN_FUEL_SECONDARY_OUTPUT
      settings: AttributesFormatString=FUNCTION=%<FUNCTION>
  ErrorCode32 : MX_Library/ErrorCode  FUNCTION=FN_THROTTLE_CONTROLLER
      settings: AttributesFormatString=FUNCTION=%<FUNCTION>
  ErrorCode32_2 : MX_Library/ErrorCode  FUNCTION=FN_THROTTLE_CONTROLLER
      settings: AttributesFormatString=FUNCTION=%<FUNCTION>
  ErrorCode32_3 : MX_Library/ErrorCode  FUNCTION=FN_THROTTLE_CONTROLLER
      settings: AttributesFormatString=FUNCTION=%<FUNCTION>
  ErrorCode33 : MX_Library/ErrorCode  FUNCTION=FN_DELUXE_PANEL_G5
      settings: AttributesFormatString=FUNCTION=%<FUNCTION>
  ErrorCode34 : MX_Library/ErrorCode  FUNCTION=FN_SWITCHED_POWER_RELAY
      settings: AttributesFormatString=FUNCTION=%<FUNCTION>
  ErrorCode35 : MX_Library/ErrorCode  FUNCTION=FN_TWO_SPEED
      settings: AttributesFormatString=FUNCTION=%<FUNCTION>
  ErrorCode36 : MX_Library/ErrorCode  FUNCTION=FN_HYD_EXCHANGE
      settings: AttributesFormatString=FUNCTION=%<FUNCTION>
  ErrorCode37 : MX_Library/ErrorCode  FUNCTION=FN_ECU_OUTPUT
      settings: AttributesFormatString=FUNCTION=%<FUNCTION>
  ErrorCode38 : MX_Library/ErrorCode  FUNCTION=FN_HVAC_OUTPUT
      settings: AttributesFormatString=FUNCTION=%<FUNCTION>
  ErrorCode39 : MX_Library/ErrorCode  FUNCTION=FN_DUMP_VALVE
      settings: AttributesFormatString=FUNCTION=%<FUNCTION>
  ErrorCode4 : MX_Library/ErrorCode  FUNCTION=FN_KEY_SWITCH
      settings: AttributesFormatString=FUNCTION=%<FUNCTION>
  ErrorCode40 : MX_Library/ErrorCode  FUNCTION=FN_HP_MATCH
      settings: AttributesFormatString=FUNCTION=%<FUNCTION>
  ErrorCode41 : MX_Library/ErrorCode  FUNCTION=FN_AUX_ROD
      settings: AttributesFormatString=FUNCTION=%<FUNCTION>
  ErrorCode42 : MX_Library/ErrorCode  FUNCTION=FN_OFFSET_BASE
      settings: AttributesFormatString=FUNCTION=%<FUNCTION>
  ErrorCode43 : MX_Library/ErrorCode  FUNCTION=FN_OFFSET_ROD
      settings: AttributesFormatString=FUNCTION=%<FUNCTION>
  ErrorCode44 : MX_Library/ErrorCode  FUNCTION=FN_KEYLESS_ENTRY
      settings: AttributesFormatString=FUNCTION=%<FUNCTION>
  ErrorCode45 : MX_Library/ErrorCode  FUNCTION=FN_JOG_SHUTTLE
      settings: AttributesFormatString=FUNCTION=%<FUNCTION>
  ErrorCode46 : MX_Library/ErrorCode  FUNCTION=FN_MAIN_CONTROLLER
      settings: AttributesFormatString=FUNCTION=%<FUNCTION>
  ErrorCode47 : MX_Library/ErrorCode  FUNCTION=FN_KEYLESS_PANEL
      settings: AttributesFormatString=FUNCTION=%<FUNCTION>
  ErrorCode48 : MX_Library/ErrorCode  FUNCTION=FN_DELUXE_PANEL_G5
      settings: AttributesFormatString=FUNCTION=%<FUNCTION>
  ErrorCode49 : MX_Library/ErrorCode  FUNCTION=FN_CONSOLE_SENSOR
      settings: AttributesFormatString=FUNCTION=%<FUNCTION>
  ErrorCode5 : MX_Library/ErrorCode  FUNCTION=FN_CONSOLE_SENSOR
      settings: AttributesFormatString=FUNCTION=%<FUNCTION>
  ErrorCode50 : MX_Library/ErrorCode  FUNCTION=FN_SECONDARY
      settings: AttributesFormatString=FUNCTION=%<FUNCTION>
  ErrorCode51 : MX_Library/ErrorCode  FUNCTION=FN_ECU_FUNCTION
      settings: AttributesFormatString=FUNCTION=%<FUNCTION>
  ErrorCode54 : MX_Library/ErrorCode  FUNCTION=FN_ENGINE_SPEED_DERATE
      settings: AttributesFormatString=FUNCTION=%<FUNCTION>
  ErrorCode55 : MX_Library/ErrorCode  FUNCTION=FN_AUX_BASE
      settings: AttributesFormatString=FUNCTION=%<FUNCTION>
  ErrorCode57 : MX_Library/ErrorCode  FUNCTION=FN_AUX_CONTROL
      settings: AttributesFormatString=FUNCTION=%<FUNCTION>
  ErrorCode58 : MX_Library/ErrorCode  FUNCTION=FN_STANDARD_DISPLAY_PANEL
      settings: AttributesFormatString=FUNCTION=%<FUNCTION>
  ErrorCode6 : MX_Library/ErrorCode  FUNCTION=FN_AIR_FILTER
      settings: AttributesFormatString=FUNCTION=%<FUNCTION>
  ErrorCode60 : MX_Library/ErrorCode  FUNCTION=FN_LEFT_CONTROL
      settings: AttributesFormatString=FUNCTION=%<FUNCTION>
  ErrorCode61 : MX_Library/ErrorCode  FUNCTION=FN_RIGHT_CONTROL
      settings: AttributesFormatString=FUNCTION=%<FUNCTION>
  ErrorCode63 : MX_Library/ErrorCode  FUNCTION=FN_TURBO_PRIME
      settings: AttributesFormatString=FUNCTION=%<FUNCTION>
  ErrorCode7 : MX_Library/ErrorCode  FUNCTION=FN_IMPLEMENT_FILTER
      settings: AttributesFormatString=FUNCTION=%<FUNCTION>
  ErrorCode70 : MX_Library/ErrorCode  FUNCTION=FN_SWITCHED_POWER_OUTPUT
      settings: AttributesFormatString=FUNCTION=%<FUNCTION>
  ErrorCode8 : MX_Library/ErrorCode  FUNCTION=FN_ENGINE_SPEED
      settings: AttributesFormatString=FUNCTION=%<FUNCTION>
  ErrorCode9 : MX_Library/ErrorCode  FUNCTION=FN_ENGINE_COOLANT_TEMPERATURE
      settings: AttributesFormatString=FUNCTION=%<FUNCTION>
  Mux : Mux
  Mux1 : Mux  Inputs=21
  Mux2 : Mux  Inputs=25
  Mux3 : Mux  Inputs=5
  Mux4 : Mux  Inputs=11
  Rate Transition{1..3,5..16,18..20,22,24} (20 alike) : RateTransition  Integrity=off
  Throttle Cal State : Stateflow chart  TreatAsAtomicUnit=on
  ECodes : Outport
  wires:
  bus_Internal_Input/1 -> Bus Selector/1
  Bus Selector/1 -> Rate Transition22/1  "<state_VBattery>"
  Bus Selector/2 -> Rate Transition1/1  "<state_Alternator>"
  Bus Selector/3 -> Rate Transition2/1  "<state_SensorSupply1>"
  Bus Selector/4 -> Rate Transition3/1  "<state_SensorSupply2>"
  Bus Selector/5 -> ErrorCode4/1  "<state_Key>"
  Bus Selector/6 -> ErrorCode5/1  "<state_Console>"
  Bus Selector/7 -> ErrorCode6/1  "<state_AirFilter>"
  Bus Selector/8 -> Rate Transition5/1  "<state_HCFilter1>"
  Bus Selector/9 -> ErrorCode8/1  "<state_EngineSpeed>"
  Bus Selector/10 -> Rate Transition7/1  "<state_ECT>"
  Bus Selector/11 -> Rate Transition8/1  "<state_HOT>"
  Bus Selector/12 -> Rate Transition9/1  "<state_EOP>"
  Bus Selector/13 -> Rate Transition10/1  "<state_FuelLevel>"
  bus_Output/1 -> Bus Selector1/1  "bus_Output"
  Bus Selector1/1 -> Rate Transition12/1  "<state_LightsOutput>"
  Bus Selector1/2 -> ErrorCode25/1  "<state_StarterOutput>"
  Bus Selector1/3 -> ErrorCode26/1  "<state_StarterRelay>"
  Bus Selector1/4 -> ErrorCode27/1  "<state_FuelPriOutput>"
  Bus Selector1/5 -> ErrorCode24/1  "<state_FuelPriRelay>"
  Bus Selector1/6 -> Rate Transition18/1  "<state_GlowPlugOutput>"
  Bus Selector1/7 -> Rate Transition19/1  "<state_GlowPlugRelay>"
  Bus Selector1/8 -> ErrorCode31/1  "<state_FuelSec>"
  Bus Selector1/9 -> ErrorCode17/1  "<state_HydEnable>"
  Bus Selector1/10 -> ErrorCode70/1  "<state_SwitchedPwrOutput>"
  Bus Selector1/11 -> ErrorCode34/1  "<state_SwitchedPwrRelay>"
  bus_Input/1 -> Bus Selector2/1
  Bus Selector2/1 -> ErrorCode46/1  "<state_ConstantData>"
  ErrorCode/1 -> Mux1/1
  ErrorCode1/1 -> Mux1/2
  ErrorCode2/1 -> Mux1/3
  ErrorCode3/1 -> Mux1/4
  ErrorCode4/1 -> Mux1/5
  ErrorCode5/1 -> Mux1/6
  ErrorCode46/1 -> Mux3/1
  Mux3/1 -> Mux/1
  ErrorCode21/1 -> Mux2/1
  ErrorCode25/1 -> Mux2/2
  ErrorCode26/1 -> Mux2/3
  ErrorCode27/1 -> Mux2/4
  ErrorCode24/1 -> Mux2/5
  ErrorCode29/1 -> Mux2/6
  ErrorCode30/1 -> Mux2/7
  ErrorCode31/1 -> Mux2/8
  ErrorCode17/1 -> Mux2/9
  ErrorCode70/1 -> Mux2/10
  ErrorCode34/1 -> Mux2/11
  Mux1/1 -> Mux/2
  Mux2/1 -> Mux/3
  Bus Selector1/12 -> ErrorCode35/1  "<state_TwoSpeed>"
  Bus Selector1/13 -> Rate Transition11/1  "<state_HydExchange>"
  ErrorCode36/1 -> Mux2/13
  Bus Selector2/2 -> ErrorCode47/1  "<state_KeylessPanel>"
  ErrorCode47/1 -> Mux3/2
  Rate Transition22/1 -> ErrorCode/1
  Rate Transition1/1 -> ErrorCode1/1
  Rate Transition2/1 -> ErrorCode2/1
  Rate Transition3/1 -> ErrorCode3/1
  Rate Transition5/1 -> ErrorCode7/1
  Rate Transition7/1 -> ErrorCode9/1
  Rate Transition8/1 -> ErrorCode10/1
  Rate Transition9/1 -> ErrorCode11/1
  Rate Transition10/1 -> ErrorCode12/1
  Rate Transition12/1 -> ErrorCode21/1
  Rate Transition18/1 -> ErrorCode29/1
  Rate Transition19/1 -> ErrorCode30/1
  ErrorCode48/1 -> Mux3/3
  ErrorCode50/1 -> Mux4/1
  bus_System/1 -> Bus Selector3/1  "bus_System"
  Bus Selector3/1 -> ErrorCode50/1  "<state_CommSecondary>"
  Bus Selector/14 -> Rate Transition24/1  "<state_ThrottlePrimary>"
  Rate Transition24/1 -> ErrorCode13/1
  Bus Selector1/14 -> ErrorCode37/1  "<state_ECUHold>"
  ErrorCode37/1 -> Mux2/14
  Bus Selector3/2 -> ErrorCode51/1  "<state_CommECU>"
  ErrorCode51/1 -> Mux4/2
  ErrorCode6/1 -> Mux1/7
  ErrorCode7/1 -> Mux1/8
  ErrorCode8/1 -> Mux1/9
  ErrorCode9/1 -> Mux1/10
  ErrorCode10/1 -> Mux1/11
  ErrorCode11/1 -> Mux1/12
  ErrorCode12/1 -> Mux1/13
  ErrorCode13/1 -> Mux1/14
  ErrorCode49/1 -> Mux3/4
  Bus Selector1/15 -> ErrorCode38/1  "<state_HVACOutput>"
  Bus Selector1/16 -> ErrorCode39/1  "<state_DumpValve>"
  ErrorCode38/1 -> Mux2/15
  ErrorCode39/1 -> Mux2/16
  ErrorCode35/1 -> Mux2/12
  ErrorCode57/1 -> Mux1/15
  Bus Selector/15 -> ErrorCode57/1  "<state_AuxPot>"
  ErrorCode18/1 -> Mux1/16
  Bus Selector/16 -> Rate Transition6/1  "<state_LoadMoment>"
  Rate Transition6/1 -> ErrorCode18/1
  Rate Transition11/1 -> ErrorCode36/1
  Bus Selector/18 -> ErrorCode20/1  "<state_LoadSense>"
  ErrorCode19/1 -> Mux1/17
  ErrorCode20/1 -> Mux1/18
  Bus Selector1/17 -> ErrorCode55/1  "<state_AuxBase>"
  Bus Selector1/18 -> ErrorCode41/1  "<state_AuxRod>"
  Bus Selector1/19 -> ErrorCode42/1  "<state_OffsetBase>"
  Bus Selector1/20 -> ErrorCode43/1  "<state_OffsetRod>"
  ErrorCode55/1 -> Mux2/17
  ErrorCode41/1 -> Mux2/18
  ErrorCode42/1 -> Mux2/19
  ErrorCode43/1 -> Mux2/20
  Bus Selector/17 -> ErrorCode19/1  "<state_OffsetPot>"
  ErrorCode32_2/1 -> Mux4/3
  Bus Selector3/3 -> ErrorCode32_2/1  "<state_CommThrottle>"
  Bus Selector3/4 -> ErrorCode54/1  "<state_EngineSpeedDerate>"
  ErrorCode54/1 -> Mux4/4
  ErrorCode14/1 -> Mux2/21
  Bus Selector1/21 -> ErrorCode14/1  "<state_HydBypass>"
  ErrorCode23/1 -> Mux2/22
  Bus Selector1/22 -> Rate Transition14/1  "<state_LightsRelay>"
  Rate Transition14/1 -> ErrorCode23/1
  Throttle Cal State/1(state_ThrottleCal) -> ErrorCode32/1
  ErrorCode32_3/1 -> Mux4/5
  Rate Transition15/1 -> ErrorCode32_3/1
  Mux4/1 -> Mux/4
  ErrorCode15/1 -> Mux1/19
  Bus Selector/19 -> Rate Transition13/1  "<state_FuelTemp>"
  Rate Transition13/1 -> ErrorCode15/1
  ErrorCode16/1 -> Mux2/23
  ErrorCode22/1 -> Mux2/24
  Bus Selector1/23 -> Rate Transition16/1  "<state_PBValveOutput>"
  Bus Selector1/24 -> Rate Transition20/1  "<state_PBValveRelay>"
  Rate Transition16/1 -> ErrorCode16/1
  Rate Transition20/1 -> ErrorCode22/1
  Mux/1 -> ECodes/1
  ErrorCode32/1 -> Mux3/5
  ErrorCode28/1 -> Mux2/25
  Bus Selector1/25 -> ErrorCode28/1  "<state_Diverter>"
  Bus Selector2/3 -> ErrorCode48/1  "<state_DeluxeG5>"
  Bus Selector2/4 -> ErrorCode49/1  "<state_ConsoleTest>"
  Bus Selector2/5 -> Throttle Cal State/1(flag_InitEERead)  "<flag_InitEERead>"
  Bus Selector3/5 -> Rate Transition15/1  "<state_CANThrottle>"
  ErrorCode40/1 -> Mux4/6
  Bus Selector3/6 -> ErrorCode40/1  "<state_HPMatch>"
  ErrorCode33/1 -> Mux4/9
  ErrorCode45/1 -> Mux4/8
  ErrorCode58/1 -> Mux4/7
  Bus Selector3/7 -> ErrorCode58/1  "<state_CommStdPanel>"
  Bus Selector3/8 -> ErrorCode45/1  "<state_CommJogShuttle>"
  Bus Selector3/9 -> ErrorCode33/1  "<state_CommDeluxeG55>"
  Bus Selector3/10 -> ErrorCode44/1  "<state_CommKeylessEntry>"
  ErrorCode44/1 -> Mux4/10
  ErrorCode61/1 -> Mux1/20
  Bus Selector/20 -> ErrorCode61/1  "<state_RightPot>"
  ErrorCode60/1 -> Mux1/21
  Bus Selector/21 -> ErrorCode60/1  "<state_LeftPot>"
  ErrorCode63/1 -> Mux4/11
  Bus Selector3/11 -> ErrorCode63/1  "<state_TurboPrime>"
